simulink library "ee_lib_for_power_systems"  (R2025b)
settings: Solver=VariableStepAuto  StartTime=0.0  StopTime=10.0  RelTol=1e-3
undefined symbols (not defined in the file or in its folder): Alt_PolePair_Count, C3m, DiscIntNeverNeededParam, Enum1to12, L3m, Total_SM_Inertia, Ts
libraries: fl_lib x291, nesl_utility x219, ee_lib x139, custom_lib x12, $bdroot x10, ee_sl_lib x3, eeTransforms x2, eeGeneralControl x1, simulink x1
(part 10 of 11)

== system ee_lib_for_power_systems/Network Components/Bergeron Line (Pos-Zero)/tau ==
  u : Inport
  Delay : Delay  DelayLength=floor(tau(1)/Ts)  SampleTime=Ts
  Delay1 : Delay  DelayLength=floor(tau(2)/Ts)  SampleTime=Ts
  Delay2 : Delay  DelayLength=floor(tau(3)/Ts)  SampleTime=Ts
  Gain : Gain  Gain=[[tau/Ts - floor(tau/Ts)]' [tau/Ts - floor(tau/Ts)]']
  Matrix Concatenate : Concatenate  NumInputs=3  Mode=Multidimensional array  ConcatenateDimension=2
  Reshape : Reshape
  Selector : Selector  InputPortWidth=6  IndexOptions=Index vector (dialog)  Indices=[1 4]  OutputSizes=1
  Selector1 : Selector  InputPortWidth=6  IndexOptions=Index vector (dialog)  Indices=[2 5]  OutputSizes=1
  Selector2 : Selector  InputPortWidth=6  IndexOptions=Index vector (dialog)  Indices=[3 6]  OutputSizes=1
  Sum : Sum  Inputs=|-+
  Sum1 : Sum  Inputs=+-|
  Transpose : Math  Operator=transpose
  Unit Delay2 : UnitDelay  SampleTime=Ts  HasFrameUpgradeWarning=on
  y : Outport
  wires:
  Sum1/1 -> y/1
  Gain/1 -> Sum1/2
  Sum/1 -> Gain/1
  u/1 -> Reshape/1
  Unit Delay2/1 -> Sum/1
  Matrix Concatenate/1 -> Transpose/1
  Delay1/1 -> Matrix Concatenate/2
  Delay/1 -> Matrix Concatenate/1
  Delay2/1 -> Matrix Concatenate/3
  Selector/1 -> Delay/1
  Selector1/1 -> Delay1/1
  Reshape/1 -> Selector2/1, Selector/1, Selector1/1
  Selector2/1 -> Delay2/1
  Transpose/1 -> Sum/2, Unit Delay2/1, Sum1/1

== system ee_lib_for_power_systems/Network Components/Bergeron Line (Pos-Zero)/tau  ==
  u : Inport
  Delay : Delay  DelayLength=floor(tau(1)/Ts)  SampleTime=Ts
  Delay1 : Delay  DelayLength=floor(tau(2)/Ts)  SampleTime=Ts
  Delay2 : Delay  DelayLength=floor(tau(3)/Ts)  SampleTime=Ts
  Gain : Gain  Gain=[[tau/Ts - floor(tau/Ts)]' [tau/Ts - floor(tau/Ts)]']
  Matrix Concatenate : Concatenate  NumInputs=3  Mode=Multidimensional array  ConcatenateDimension=2
  Reshape : Reshape
  Selector : Selector  InputPortWidth=6  IndexOptions=Index vector (dialog)  Indices=[1 4]  OutputSizes=1
  Selector1 : Selector  InputPortWidth=6  IndexOptions=Index vector (dialog)  Indices=[2 5]  OutputSizes=1
  Selector2 : Selector  InputPortWidth=6  IndexOptions=Index vector (dialog)  Indices=[3 6]  OutputSizes=1
  Sum : Sum  Inputs=|-+
  Sum1 : Sum  Inputs=+-|
  Transpose : Math  Operator=transpose
  Unit Delay2 : UnitDelay  SampleTime=Ts  HasFrameUpgradeWarning=on
  y : Outport
  wires:
  Transpose/1 -> Sum1/1, Unit Delay2/1, Sum/2
  Selector2/1 -> Delay2/1
  Reshape/1 -> Selector1/1, Selector/1, Selector2/1
  Selector1/1 -> Delay1/1
  Selector/1 -> Delay/1
  Delay2/1 -> Matrix Concatenate/3
  Delay/1 -> Matrix Concatenate/1
  Delay1/1 -> Matrix Concatenate/2
  Matrix Concatenate/1 -> Transpose/1
  Unit Delay2/1 -> Sum/1
  u/1 -> Reshape/1
  Sum/1 -> Gain/1
  Gain/1 -> Sum1/2
  Sum1/1 -> y/1

== system ee_lib_for_power_systems/Network Components/Bergeron Line (single-phase)/tau ==
  u : Inport
  Delay : Delay  DelayLength=floor(tau/Ts)  SampleTime=Ts
  Gain : Gain  Gain=tau/Ts - floor(tau/Ts)
  Sum : Sum  Inputs=|-+
  Sum1 : Sum  Inputs=+-|
  Unit Delay2 : UnitDelay  SampleTime=Ts  HasFrameUpgradeWarning=on
  y : Outport
  wires:
  Delay/1 -> Sum1/1, Unit Delay2/1, Sum/2
  u/1 -> Delay/1
  Unit Delay2/1 -> Sum/1
  Sum/1 -> Gain/1
  Gain/1 -> Sum1/2
  Sum1/1 -> y/1

== system ee_lib_for_power_systems/Network Components/Bergeron Line (single-phase)/tau  ==
  u : Inport
  Delay : Delay  DelayLength=floor(tau/Ts)  SampleTime=Ts
  Gain : Gain  Gain=tau/Ts - floor(tau/Ts)
  Sum : Sum  Inputs=|-+
  Sum1 : Sum  Inputs=+-|
  Unit Delay2 : UnitDelay  SampleTime=Ts  HasFrameUpgradeWarning=on
  y : Outport
  wires:
  Sum1/1 -> y/1
  Gain/1 -> Sum1/2
  Sum/1 -> Gain/1
  Unit Delay2/1 -> Sum/1
  u/1 -> Delay/1
  Delay/1 -> Sum/2, Unit Delay2/1, Sum1/1

== system ee_lib_for_power_systems/Network Components/Connector Bergeron Line (Pos-Zero)/tau ==
  u : Inport
  Delay : Delay  DelayLength=floor(tau(1)/Ts)  SampleTime=Ts
  Delay1 : Delay  DelayLength=floor(tau(2)/Ts)  SampleTime=Ts
  Delay2 : Delay  DelayLength=floor(tau(3)/Ts)  SampleTime=Ts
  Gain : Gain  Gain=[tau/Ts - floor(tau/Ts)]'
  Matrix Concatenate : Concatenate  NumInputs=3  Mode=Multidimensional array  ConcatenateDimension=2
  Reshape : Reshape
  Selector : Selector  InputPortWidth=3  IndexOptions=Index vector (dialog)  Indices=1  OutputSizes=1
  Selector1 : Selector  InputPortWidth=3  IndexOptions=Index vector (dialog)  Indices=2  OutputSizes=1
  Selector2 : Selector  InputPortWidth=3  IndexOptions=Index vector (dialog)  Indices=3  OutputSizes=1
  Sum : Sum  Inputs=|-+
  Sum1 : Sum  Inputs=+-|
  Transpose : Math  Operator=transpose
  Unit Delay2 : UnitDelay  SampleTime=Ts  HasFrameUpgradeWarning=on
  y : Outport
  wires:
  Sum1/1 -> y/1
  Gain/1 -> Sum1/2
  Sum/1 -> Gain/1
  u/1 -> Reshape/1
  Unit Delay2/1 -> Sum/1
  Matrix Concatenate/1 -> Transpose/1
  Delay1/1 -> Matrix Concatenate/2
  Delay/1 -> Matrix Concatenate/1
  Delay2/1 -> Matrix Concatenate/3
  Selector/1 -> Delay/1
  Selector1/1 -> Delay1/1
  Reshape/1 -> Selector2/1, Selector1/1, Selector/1
  Selector2/1 -> Delay2/1
  Transpose/1 -> Sum/2, Unit Delay2/1, Sum1/1

== system ee_lib_for_power_systems/Network Components/Connector Bergeron Line (Pos-Zero)/tau  ==
  u : Inport
  Delay : Delay  DelayLength=floor(tau(1)/Ts)  SampleTime=Ts
  Delay1 : Delay  DelayLength=floor(tau(2)/Ts)  SampleTime=Ts
  Delay2 : Delay  DelayLength=floor(tau(3)/Ts)  SampleTime=Ts
  Gain : Gain  Gain=[tau/Ts - floor(tau/Ts)]'
  Matrix Concatenate : Concatenate  NumInputs=3  Mode=Multidimensional array  ConcatenateDimension=2
  Reshape : Reshape
  Selector : Selector  InputPortWidth=3  IndexOptions=Index vector (dialog)  Indices=1  OutputSizes=1
  Selector1 : Selector  InputPortWidth=3  IndexOptions=Index vector (dialog)  Indices=2  OutputSizes=1
  Selector2 : Selector  InputPortWidth=3  IndexOptions=Index vector (dialog)  Indices=3  OutputSizes=1
  Sum : Sum  Inputs=|-+
  Sum1 : Sum  Inputs=+-|
  Transpose : Math  Operator=transpose
  Unit Delay2 : UnitDelay  SampleTime=Ts  HasFrameUpgradeWarning=on
  y : Outport
  wires:
  Transpose/1 -> Sum1/1, Unit Delay2/1, Sum/2
  Selector2/1 -> Delay2/1
  Reshape/1 -> Selector/1, Selector1/1, Selector2/1
  Selector1/1 -> Delay1/1
  Selector/1 -> Delay/1
  Delay2/1 -> Matrix Concatenate/3
  Delay/1 -> Matrix Concatenate/1
  Delay1/1 -> Matrix Concatenate/2
  Matrix Concatenate/1 -> Transpose/1
  Unit Delay2/1 -> Sum/1
  u/1 -> Reshape/1
  Sum/1 -> Gain/1
  Gain/1 -> Sum1/2
  Sum1/1 -> y/1

== system ee_lib_for_power_systems/Network Components/Connector Bergeron Line (single-phase)/tau ==
  u : Inport
  Delay : Delay  DelayLength=floor(tau/Ts)  SampleTime=Ts
  Gain : Gain  Gain=tau/Ts - floor(tau/Ts)
  Sum : Sum  Inputs=|-+
  Sum1 : Sum  Inputs=+-|
  Unit Delay2 : UnitDelay  SampleTime=Ts  HasFrameUpgradeWarning=on
  y : Outport
  wires:
  Delay/1 -> Sum1/1, Unit Delay2/1, Sum/2
  u/1 -> Delay/1
  Unit Delay2/1 -> Sum/1
  Sum/1 -> Gain/1
  Gain/1 -> Sum1/2
  Sum1/1 -> y/1

== system ee_lib_for_power_systems/Network Components/Connector Bergeron Line (single-phase)/tau  ==
  u : Inport
  Delay : Delay  DelayLength=floor(tau/Ts)  SampleTime=Ts
  Gain : Gain  Gain=tau/Ts - floor(tau/Ts)
  Sum : Sum  Inputs=|-+
  Sum1 : Sum  Inputs=+-|
  Unit Delay2 : UnitDelay  SampleTime=Ts  HasFrameUpgradeWarning=on
  y : Outport
  wires:
  Sum1/1 -> y/1
  Gain/1 -> Sum1/2
  Sum/1 -> Gain/1
  Unit Delay2/1 -> Sum/1
  u/1 -> Delay/1
  Delay/1 -> Sum/2, Unit Delay2/1, Sum1/1

== system ee_lib_for_power_systems/Power Converters/Ideal Switch Basic Diode Two-Level/S1 ==
  note: orientation based on anti-parallel diode
  g : Inport
  Ideal Switch with Basic Diode : custom_lib/Ideal Switch with Basic Diode  Ron=Ron Ohm  Goff=Goff 1/Ohm  i_specify=off  i_priority=None  i=0 A  i_nominal_specify=off  i_nominal_value=1  i_nominal_unit=A  v_specify=off  v_priority=None  v=0 V  v_nominal_specify=off  v_nominal_value=1  v_nominal_unit=V
      settings: InternalSimscapePortConfiguration="{\"Bottom\":[],\"Left\":[{\"id\":\"p\",\"label\":\"+\",\"type\":\"foundation.electrical.electrical\"},{\"id\":\"vT\",\"label\":\"\",\"type\":\"input\"}],\"Right\":[{\"id\":\"n\",\"label\":\"-\",\"type\":\"foundation.electrical.electrical\"}],\"Top\":[]}"
  Simulink-PS Converter : nesl_utility/Simulink-PS Converter  PhysicalDomain=network_engine_domain  SubClassName=ps_input  LeftPortType=input  RightPortType=output  PseudoPeriodic=off  Frequencies=[]  NoiseDistribution=none  NoiseParameters=[]  Unit=1  AffineConversion=off  FilteringAndDerivatives=provide  SimscapeFilterOrder=1  InputFilterTimeConstant=0.001  UdotUserProvided=0
  + : PMIOPort  Side=Left
  - : PMIOPort  Port=2  Side=Right
  wires:
  g/1 -> Simulink-PS Converter/1
  connections:
  Ideal Switch with Basic Diode/LConn1 -- -/RConn1
  Ideal Switch with Basic Diode/RConn1 -- +/RConn1
  Ideal Switch with Basic Diode/LConn2 -- Simulink-PS Converter/RConn1

== system ee_lib_for_power_systems/Power Converters/Three-Level Average-Value/Subsystem ==
  open : Inport
  Current Sensor : fl_lib/Electrical/Electrical Sensors/Current Sensor
  Data Type Conversion : DataTypeConversion  SaturateOnIntegerOverflow=off  RndMeth=Floor
  Ideal Semiconductor Switch{,1..3} (4 alike) : ee_lib/Semiconductors & Converters/Ideal Semiconductor Switch  port_option=ee.enum.controlport.ps  Ron=1e-8 Ohm  Goff=1e-8 1/Ohm  Vth=0.5 V  diode_param=ee.enum.semiconductors.protectionDiode.external  diode_Vf=0.8 V  diode_Ron=0.001 Ohm  diode_Goff=1e-5 1/Ohm  diode_C=50e-9 F  diode_iRM=-235 A  diode_iF=300 A  diode_idot=-50 A/us  diode_trr_param=ee.enum.converters.diodetrr.trr_factor  diode_trr_factor=3  diode_trr=15 us  diode_Qrr=1500 s*uA
  NOT : Logic  Operator=NOT  IconShape=distinctive  AllPortsSameDT=off  OutDataTypeStr=boolean
  Simulink-PS Converter2 : nesl_utility/Simulink-PS Converter  PhysicalDomain=network_engine_domain  SubClassName=ps_input  LeftPortType=input  RightPortType=output  PseudoPeriodic=off  Frequencies=[]  NoiseDistribution=none  NoiseParameters=[]  Unit=1  AffineConversion=off  FilteringAndDerivatives=provide  SimscapeFilterOrder=1  InputFilterTimeConstant=0.001  UdotUserProvided=0
  Simulink-PS Converter3 : nesl_utility/Simulink-PS Converter  PhysicalDomain=network_engine_domain  SubClassName=ps_input  LeftPortType=input  RightPortType=output  PseudoPeriodic=off  Frequencies=[]  NoiseDistribution=none  NoiseParameters=[]  Unit=1  AffineConversion=off  FilteringAndDerivatives=provide  SimscapeFilterOrder=1  InputFilterTimeConstant=0.001  UdotUserProvided=0
  N : PMIOPort  Side=Left
  +1 : PMIOPort  Port=2  Side=Left
  +2 : PMIOPort  Port=3  Side=Left
  Iconn : PMIOPort  Port=4  Side=Right
  -1 : PMIOPort  Port=5  Side=Right
  -2 : PMIOPort  Port=6  Side=Right
  wires:
  open/1 -> Simulink-PS Converter2/1, NOT/1
  NOT/1 -> Data Type Conversion/1
  Data Type Conversion/1 -> Simulink-PS Converter3/1
  connections:
  Current Sensor/RConn2 -- Ideal Semiconductor Switch1/RConn1 -- Ideal Semiconductor Switch2/RConn2
  Iconn/RConn1 -- Current Sensor/RConn1
  Current Sensor/LConn1 -- N/RConn1
  Ideal Semiconductor Switch2/LConn1 -- Simulink-PS Converter3/RConn1 -- Ideal Semiconductor Switch1/LConn1
  +2/RConn1 -- Ideal Semiconductor Switch/RConn1 -- Ideal Semiconductor Switch1/RConn2
  Ideal Semiconductor Switch/LConn1 -- Simulink-PS Converter2/RConn1 -- Ideal Semiconductor Switch3/LConn1
  Ideal Semiconductor Switch/RConn2 -- +1/RConn1
  Ideal Semiconductor Switch3/RConn1 -- Ideal Semiconductor Switch2/RConn1 -- -2/RConn1
  -1/RConn1 -- Ideal Semiconductor Switch3/RConn2

== system ee_lib_for_power_systems/Power Converters/Three-Level Average-Value/Subsystem1 ==
  open : Inport
  g_h : Inport  Port=2
  Constant1 : Constant  Value=0  SampleTime=-1
  Switch : Switch  Criteria=u2 > Threshold  Threshold=0.5  SaturateOnIntegerOverflow=off  InputSameDT=off
  p : Outport
  wires:
  g_h/1 -> Switch/3
  open/1 -> Switch/2
  Constant1/1 -> Switch/1
  Switch/1 -> p/1

== system ee_lib_for_power_systems/Power Converters/Three-Level Average-Value/Subsystem2 ==
  p3 : Inport
  p2 : Inport  Port=2
  p1 : Inport  Port=3
  PS Add : fl_lib/Physical Signals/Functions/PS Add
  PS Add1 : fl_lib/Physical Signals/Functions/PS Add
  PS Gain : fl_lib/Physical Signals/Functions/PS Gain  gain=-1
  PS Product2 : fl_lib/Physical Signals/Functions/PS Product
  PS Product7 : fl_lib/Physical Signals/Functions/PS Product
  PS Product8 : fl_lib/Physical Signals/Functions/PS Product
  Simulink-PS Converter : nesl_utility/Simulink-PS Converter  PhysicalDomain=network_engine_domain  SubClassName=ps_input  LeftPortType=input  RightPortType=output  PseudoPeriodic=off  Frequencies=[]  NoiseDistribution=none  NoiseParameters=[]  Unit=1  AffineConversion=off  FilteringAndDerivatives=provide  SimscapeFilterOrder=1  InputFilterTimeConstant=0.001  UdotUserProvided=0
  Simulink-PS Converter1 : nesl_utility/Simulink-PS Converter  PhysicalDomain=network_engine_domain  SubClassName=ps_input  LeftPortType=input  RightPortType=output  PseudoPeriodic=off  Frequencies=[]  NoiseDistribution=none  NoiseParameters=[]  Unit=1  AffineConversion=off  FilteringAndDerivatives=provide  SimscapeFilterOrder=1  InputFilterTimeConstant=0.001  UdotUserProvided=0
  Simulink-PS Converter2 : nesl_utility/Simulink-PS Converter  PhysicalDomain=network_engine_domain  SubClassName=ps_input  LeftPortType=input  RightPortType=output  PseudoPeriodic=off  Frequencies=[]  NoiseDistribution=none  NoiseParameters=[]  Unit=1  AffineConversion=off  FilteringAndDerivatives=provide  SimscapeFilterOrder=1  InputFilterTimeConstant=0.001  UdotUserProvided=0
  IAP : PMIOPort  Side=Left
  IBP : PMIOPort  Port=2  Side=Left
  ICP : PMIOPort  Port=3  Side=Left
  IDCP : PMIOPort  Port=4  Side=Right
  wires:
  p3/1 -> Simulink-PS Converter2/1
  p2/1 -> Simulink-PS Converter1/1
  p1/1 -> Simulink-PS Converter/1
  connections:
  PS Gain/LConn1 -- PS Add1/RConn1
  PS Gain/RConn1 -- IDCP/RConn1
  PS Product2/LConn1 -- IAP/RConn1
  PS Add1/LConn1 -- PS Product8/RConn1
  PS Add1/LConn2 -- PS Add/RConn1
  Simulink-PS Converter/RConn1 -- PS Product2/LConn2
  PS Add/LConn2 -- PS Product2/RConn1
  PS Add/LConn1 -- PS Product7/RConn1
  PS Product8/LConn1 -- ICP/RConn1
  PS Product8/LConn2 -- Simulink-PS Converter2/RConn1
  PS Product7/LConn2 -- Simulink-PS Converter1/RConn1
  PS Product7/LConn1 -- IBP/RConn1

== system ee_lib_for_power_systems/Power Converters/Three-Level Average-Value/Subsystem4 ==
  p3 : Inport
  p2 : Inport  Port=2
  p1 : Inport  Port=3
  PS Add : fl_lib/Physical Signals/Functions/PS Add
  PS Add1 : fl_lib/Physical Signals/Functions/PS Add
  PS Gain : fl_lib/Physical Signals/Functions/PS Gain  gain=1
  PS Product2 : fl_lib/Physical Signals/Functions/PS Product
  PS Product7 : fl_lib/Physical Signals/Functions/PS Product
  PS Product8 : fl_lib/Physical Signals/Functions/PS Product
  Simulink-PS Converter : nesl_utility/Simulink-PS Converter  PhysicalDomain=network_engine_domain  SubClassName=ps_input  LeftPortType=input  RightPortType=output  PseudoPeriodic=off  Frequencies=[]  NoiseDistribution=none  NoiseParameters=[]  Unit=1  AffineConversion=off  FilteringAndDerivatives=provide  SimscapeFilterOrder=1  InputFilterTimeConstant=0.001  UdotUserProvided=0
  Simulink-PS Converter1 : nesl_utility/Simulink-PS Converter  PhysicalDomain=network_engine_domain  SubClassName=ps_input  LeftPortType=input  RightPortType=output  PseudoPeriodic=off  Frequencies=[]  NoiseDistribution=none  NoiseParameters=[]  Unit=1  AffineConversion=off  FilteringAndDerivatives=provide  SimscapeFilterOrder=1  InputFilterTimeConstant=0.001  UdotUserProvided=0
  Simulink-PS Converter2 : nesl_utility/Simulink-PS Converter  PhysicalDomain=network_engine_domain  SubClassName=ps_input  LeftPortType=input  RightPortType=output  PseudoPeriodic=off  Frequencies=[]  NoiseDistribution=none  NoiseParameters=[]  Unit=1  AffineConversion=off  FilteringAndDerivatives=provide  SimscapeFilterOrder=1  InputFilterTimeConstant=0.001  UdotUserProvided=0
  IAN : PMIOPort  Side=Left
  IBN : PMIOPort  Port=2  Side=Left
  ICN : PMIOPort  Port=3  Side=Left
  IDCN : PMIOPort  Port=4  Side=Right
  wires:
  p1/1 -> Simulink-PS Converter/1
  p2/1 -> Simulink-PS Converter1/1
  p3/1 -> Simulink-PS Converter2/1
  connections:
  PS Product7/LConn1 -- IBN/RConn1
  PS Product7/LConn2 -- Simulink-PS Converter1/RConn1
  PS Product8/LConn2 -- Simulink-PS Converter2/RConn1
  PS Product8/LConn1 -- ICN/RConn1
  PS Add/LConn1 -- PS Product7/RConn1
  PS Add/LConn2 -- PS Product2/RConn1
  Simulink-PS Converter/RConn1 -- PS Product2/LConn2
  PS Add1/LConn2 -- PS Add/RConn1
  PS Add1/LConn1 -- PS Product8/RConn1
  PS Product2/LConn1 -- IAN/RConn1
  PS Gain/RConn1 -- IDCN/RConn1
  PS Add1/RConn1 -- PS Gain/LConn1

== system ee_lib_for_power_systems/Power Converters/Three-Level NPC Average-Switch with Diodes/IDCN ==
  p3 : Inport
  p2 : Inport  Port=2
  p1 : Inport  Port=3
  PS Add : fl_lib/Physical Signals/Functions/PS Add
  PS Add1 : fl_lib/Physical Signals/Functions/PS Add
  PS Gain : fl_lib/Physical Signals/Functions/PS Gain  gain=1
  PS Product1 : fl_lib/Physical Signals/Functions/PS Product
  PS Product2 : fl_lib/Physical Signals/Functions/PS Product
  PS Product3 : fl_lib/Physical Signals/Functions/PS Product
  Simulink-PS Converter1 : nesl_utility/Simulink-PS Converter  PhysicalDomain=network_engine_domain  SubClassName=ps_input  LeftPortType=input  RightPortType=output  PseudoPeriodic=off  Frequencies=[]  NoiseDistribution=none  NoiseParameters=[]  Unit=1  AffineConversion=off  FilteringAndDerivatives=zero  SimscapeFilterOrder=1  InputFilterTimeConstant=0.001  UdotUserProvided=0
  Simulink-PS Converter2 : nesl_utility/Simulink-PS Converter  PhysicalDomain=network_engine_domain  SubClassName=ps_input  LeftPortType=input  RightPortType=output  PseudoPeriodic=off  Frequencies=[]  NoiseDistribution=none  NoiseParameters=[]  Unit=1  AffineConversion=off  FilteringAndDerivatives=zero  SimscapeFilterOrder=1  InputFilterTimeConstant=0.001  UdotUserProvided=0
  Simulink-PS Converter3 : nesl_utility/Simulink-PS Converter  PhysicalDomain=network_engine_domain  SubClassName=ps_input  LeftPortType=input  RightPortType=output  PseudoPeriodic=off  Frequencies=[]  NoiseDistribution=none  NoiseParameters=[]  Unit=1  AffineConversion=off  FilteringAndDerivatives=zero  SimscapeFilterOrder=1  InputFilterTimeConstant=0.001  UdotUserProvided=0
  IAN : PMIOPort  Side=Left
  IBN : PMIOPort  Port=2  Side=Left
  ICN : PMIOPort  Port=3  Side=Left
  IDCN : PMIOPort  Port=4  Side=Right
  wires:
  p2/1 -> Simulink-PS Converter1/1
  p3/1 -> Simulink-PS Converter3/1
  p1/1 -> Simulink-PS Converter2/1
  connections:
  PS Product1/RConn1 -- PS Add1/LConn1
  PS Add1/RConn1 -- PS Add/LConn2
  PS Product3/RConn1 -- PS Add/LConn1
  IDCN/RConn1 -- PS Gain/RConn1
  PS Gain/LConn1 -- PS Add/RConn1
  PS Product2/RConn1 -- PS Add1/LConn2
  IAN/RConn1 -- PS Product2/LConn1
  IBN/RConn1 -- PS Product1/LConn1
  ICN/RConn1 -- PS Product3/LConn1
  Simulink-PS Converter2/RConn1 -- PS Product2/LConn2
  Simulink-PS Converter1/RConn1 -- PS Product1/LConn2
  Simulink-PS Converter3/RConn1 -- PS Product3/LConn2

== system ee_lib_for_power_systems/Power Converters/Three-Level NPC Average-Switch with Diodes/IDCP ==
  p3 : Inport
  p2 : Inport  Port=2
  p1 : Inport  Port=3
  PS Add : fl_lib/Physical Signals/Functions/PS Add
  PS Add1 : fl_lib/Physical Signals/Functions/PS Add
  PS Gain : fl_lib/Physical Signals/Functions/PS Gain  gain=-1
  PS Product : fl_lib/Physical Signals/Functions/PS Product
  PS Product1 : fl_lib/Physical Signals/Functions/PS Product
  PS Product2 : fl_lib/Physical Signals/Functions/PS Product
  Simulink-PS Converter : nesl_utility/Simulink-PS Converter  PhysicalDomain=network_engine_domain  SubClassName=ps_input  LeftPortType=input  RightPortType=output  PseudoPeriodic=off  Frequencies=[]  NoiseDistribution=none  NoiseParameters=[]  Unit=1  AffineConversion=off  FilteringAndDerivatives=zero  SimscapeFilterOrder=1  InputFilterTimeConstant=0.001  UdotUserProvided=0
  Simulink-PS Converter1 : nesl_utility/Simulink-PS Converter  PhysicalDomain=network_engine_domain  SubClassName=ps_input  LeftPortType=input  RightPortType=output  PseudoPeriodic=off  Frequencies=[]  NoiseDistribution=none  NoiseParameters=[]  Unit=1  AffineConversion=off  FilteringAndDerivatives=zero  SimscapeFilterOrder=1  InputFilterTimeConstant=0.001  UdotUserProvided=0
  Simulink-PS Converter2 : nesl_utility/Simulink-PS Converter  PhysicalDomain=network_engine_domain  SubClassName=ps_input  LeftPortType=input  RightPortType=output  PseudoPeriodic=off  Frequencies=[]  NoiseDistribution=none  NoiseParameters=[]  Unit=1  AffineConversion=off  FilteringAndDerivatives=zero  SimscapeFilterOrder=1  InputFilterTimeConstant=0.001  UdotUserProvided=0
  IAP : PMIOPort  Side=Left
  IBP : PMIOPort  Port=2  Side=Left
  ICP : PMIOPort  Port=3  Side=Left
  IDCP : PMIOPort  Port=4  Side=Right
  wires:
  p1/1 -> Simulink-PS Converter/1
  p3/1 -> Simulink-PS Converter2/1
  p2/1 -> Simulink-PS Converter1/1
  connections:
  PS Gain/RConn1 -- IDCP/RConn1
  PS Add/RConn1 -- PS Gain/LConn1
  PS Product2/RConn1 -- PS Add/LConn1
  PS Add1/RConn1 -- PS Add/LConn2
  PS Product1/RConn1 -- PS Add1/LConn1
  PS Product/RConn1 -- PS Add1/LConn2
  IAP/RConn1 -- PS Product/LConn1
  IBP/RConn1 -- PS Product1/LConn1
  ICP/RConn1 -- PS Product2/LConn1
  Simulink-PS Converter/RConn1 -- PS Product/LConn2
  Simulink-PS Converter1/RConn1 -- PS Product1/LConn2
  Simulink-PS Converter2/RConn1 -- PS Product2/LConn2

== system ee_lib_for_power_systems/Power Converters/Three-Level NPC Average-Switch with Diodes/IN ==
  p3 : Inport
  p2 : Inport  Port=2
  p1 : Inport  Port=3
  PS Add : fl_lib/Physical Signals/Functions/PS Add
  PS Add1 : fl_lib/Physical Signals/Functions/PS Add
  PS Product : fl_lib/Physical Signals/Functions/PS Product
  PS Product1 : fl_lib/Physical Signals/Functions/PS Product
  PS Product2 : fl_lib/Physical Signals/Functions/PS Product
  Simulink-PS Converter : nesl_utility/Simulink-PS Converter  PhysicalDomain=network_engine_domain  SubClassName=ps_input  LeftPortType=input  RightPortType=output  PseudoPeriodic=off  Frequencies=[]  NoiseDistribution=none  NoiseParameters=[]  Unit=1  AffineConversion=off  FilteringAndDerivatives=zero  SimscapeFilterOrder=1  InputFilterTimeConstant=0.001  UdotUserProvided=0
  Simulink-PS Converter1 : nesl_utility/Simulink-PS Converter  PhysicalDomain=network_engine_domain  SubClassName=ps_input  LeftPortType=input  RightPortType=output  PseudoPeriodic=off  Frequencies=[]  NoiseDistribution=none  NoiseParameters=[]  Unit=1  AffineConversion=off  FilteringAndDerivatives=zero  SimscapeFilterOrder=1  InputFilterTimeConstant=0.001  UdotUserProvided=0
  Simulink-PS Converter2 : nesl_utility/Simulink-PS Converter  PhysicalDomain=network_engine_domain  SubClassName=ps_input  LeftPortType=input  RightPortType=output  PseudoPeriodic=off  Frequencies=[]  NoiseDistribution=none  NoiseParameters=[]  Unit=1  AffineConversion=off  FilteringAndDerivatives=zero  SimscapeFilterOrder=1  InputFilterTimeConstant=0.001  UdotUserProvided=0
  IA : PMIOPort  Side=Left
  IB : PMIOPort  Port=2  Side=Left
  IC : PMIOPort  Port=3  Side=Left
  IN : PMIOPort  Port=4  Side=Right
  wires:
  p1/1 -> Simulink-PS Converter/1
  p2/1 -> Simulink-PS Converter1/1
  p3/1 -> Simulink-PS Converter2/1
  connections:
  PS Product/RConn1 -- PS Add1/LConn2
  PS Product1/RConn1 -- PS Add1/LConn1
  PS Add1/RConn1 -- PS Add/LConn2
  PS Product2/RConn1 -- PS Add/LConn1
  PS Add/RConn1 -- IN/RConn1
  IA/RConn1 -- PS Product/LConn1
  IB/RConn1 -- PS Product1/LConn1
  IC/RConn1 -- PS Product2/LConn1
  Simulink-PS Converter/RConn1 -- PS Product/LConn2
  Simulink-PS Converter1/RConn1 -- PS Product1/LConn2
  Simulink-PS Converter2/RConn1 -- PS Product2/LConn2

== system ee_lib_for_power_systems/Power Converters/Three-Level NPC Average-Switch with Diodes/Subsystem ==
  open : Inport
  Data Type Conversion : DataTypeConversion  SaturateOnIntegerOverflow=off  RndMeth=Floor
  Ideal Semiconductor Switch{,1..3} (4 alike) : ee_lib/Semiconductors & Converters/Ideal Semiconductor Switch  port_option=ee.enum.controlport.ps  Ron=Ron Ohm  Goff=Goff 1/Ohm  Vth=0.5 V  diode_param=ee.enum.semiconductors.protectionDiode.external  diode_Vf=0.8 V  diode_Ron=0.001 Ohm  diode_Goff=1e-5 1/Ohm  diode_C=50e-9 F  diode_iRM=-235 A  diode_iF=300 A  diode_idot=-50 A/us  diode_trr_param=ee.enum.converters.diodetrr.trr_factor  diode_trr_factor=3  diode_trr=15 us  diode_Qrr=1500 s*uA
  NOT : Logic  Operator=NOT  IconShape=distinctive  AllPortsSameDT=off  OutDataTypeStr=boolean
  Simulink-PS Converter2 : nesl_utility/Simulink-PS Converter  PhysicalDomain=network_engine_domain  SubClassName=ps_input  LeftPortType=input  RightPortType=output  PseudoPeriodic=off  Frequencies=[]  NoiseDistribution=none  NoiseParameters=[]  Unit=1  AffineConversion=off  FilteringAndDerivatives=provide  SimscapeFilterOrder=1  InputFilterTimeConstant=0.001  UdotUserProvided=0
  Simulink-PS Converter3 : nesl_utility/Simulink-PS Converter  PhysicalDomain=network_engine_domain  SubClassName=ps_input  LeftPortType=input  RightPortType=output  PseudoPeriodic=off  Frequencies=[]  NoiseDistribution=none  NoiseParameters=[]  Unit=1  AffineConversion=off  FilteringAndDerivatives=provide  SimscapeFilterOrder=1  InputFilterTimeConstant=0.001  UdotUserProvided=0
  N : PMIOPort  Side=Left
  +1 : PMIOPort  Port=2  Side=Left
  +2 : PMIOPort  Port=3  Side=Left
  -1 : PMIOPort  Port=4  Side=Right
  -2 : PMIOPort  Port=5  Side=Right
  wires:
  open/1 -> Simulink-PS Converter2/1, NOT/1
  NOT/1 -> Data Type Conversion/1
  Data Type Conversion/1 -> Simulink-PS Converter3/1
  connections:
  Ideal Semiconductor Switch2/RConn2 -- Ideal Semiconductor Switch3/RConn1 -- N/RConn1
  Ideal Semiconductor Switch2/LConn1 -- Ideal Semiconductor Switch3/LConn1 -- Simulink-PS Converter3/RConn1
  +2/RConn1 -- Ideal Semiconductor Switch3/RConn2 -- Ideal Semiconductor Switch/RConn1
  Ideal Semiconductor Switch/LConn1 -- Ideal Semiconductor Switch1/LConn1 -- Simulink-PS Converter2/RConn1
  Ideal Semiconductor Switch/RConn2 -- +1/RConn1
  Ideal Semiconductor Switch1/RConn1 -- Ideal Semiconductor Switch2/RConn1 -- -2/RConn1
  Ideal Semiconductor Switch1/RConn2 -- -1/RConn1

== system ee_lib_for_power_systems/Power Converters/Three-Level NPC Average-Switch with Diodes/Subsystem1 ==
  Voltage Sensor : fl_lib/Electrical/Electrical Sensors/Voltage Sensor
  + : PMIOPort  Side=Left
  - : PMIOPort  Port=2  Side=Right
  vmeas : PMIOPort  Port=3  Side=Right
  connections:
  vmeas/RConn1 -- Voltage Sensor/RConn1
  Voltage Sensor/LConn1 -- +/RConn1
  Voltage Sensor/RConn2 -- -/RConn1

== system ee_lib_for_power_systems/Power Converters/Three-Level NPC Average-Switch with Diodes/Subsystem11 ==
  open : Inport
  g_h : Inport  Port=2
  Constant1 : Constant  Value=[0 0 0]  SampleTime=-1
  Switch : Switch  Criteria=u2 > Threshold  Threshold=0.5  SaturateOnIntegerOverflow=off  InputSameDT=off
  p : Outport
  wires:
  Switch/1 -> p/1
  Constant1/1 -> Switch/1
  open/1 -> Switch/2
  g_h/1 -> Switch/3

== system ee_lib_for_power_systems/Power Converters/Three-Level NPC Average-Switch with Diodes/Subsystem3 ==
  Voltage Sensor : fl_lib/Electrical/Electrical Sensors/Voltage Sensor
  + : PMIOPort  Side=Left
  vmeas : PMIOPort  Port=2  Side=Left
  - : PMIOPort  Port=3  Side=Right
  connections:
  Voltage Sensor/RConn2 -- -/RConn1
  Voltage Sensor/LConn1 -- +/RConn1
  vmeas/RConn1 -- Voltage Sensor/RConn1

== system ee_lib_for_power_systems/Power Converters/Three-Level NPC Average-Switch with Diodes/Subsystem4 ==
  Voltage Sensor : fl_lib/Electrical/Electrical Sensors/Voltage Sensor
  + : PMIOPort  Side=Left
  - : PMIOPort  Port=2  Side=Right
  vmeas : PMIOPort  Port=3  Side=Right
  connections:
  Voltage Sensor/RConn2 -- -/RConn1
  Voltage Sensor/LConn1 -- +/RConn1
  vmeas/RConn1 -- Voltage Sensor/RConn1

== system ee_lib_for_power_systems/Power Converters/Three-Level NPC Average-Switch with Diodes/VN ==
  p1n : Inport
  p2n : Inport  Port=2
  p3n : Inport  Port=3
  PS Product : fl_lib/Physical Signals/Functions/PS Product
  PS Product1 : fl_lib/Physical Signals/Functions/PS Product
  PS Product2 : fl_lib/Physical Signals/Functions/PS Product
  Simulink-PS Converter7 : nesl_utility/Simulink-PS Converter  PhysicalDomain=network_engine_domain  SubClassName=ps_input  LeftPortType=input  RightPortType=output  PseudoPeriodic=off  Frequencies=[]  NoiseDistribution=none  NoiseParameters=[]  Unit=1  AffineConversion=off  FilteringAndDerivatives=provide  SimscapeFilterOrder=1  InputFilterTimeConstant=0.001  UdotUserProvided=0
  Simulink-PS Converter8 : nesl_utility/Simulink-PS Converter  PhysicalDomain=network_engine_domain  SubClassName=ps_input  LeftPortType=input  RightPortType=output  PseudoPeriodic=off  Frequencies=[]  NoiseDistribution=none  NoiseParameters=[]  Unit=1  AffineConversion=off  FilteringAndDerivatives=provide  SimscapeFilterOrder=1  InputFilterTimeConstant=0.001  UdotUserProvided=0
  Simulink-PS Converter9 : nesl_utility/Simulink-PS Converter  PhysicalDomain=network_engine_domain  SubClassName=ps_input  LeftPortType=input  RightPortType=output  PseudoPeriodic=off  Frequencies=[]  NoiseDistribution=none  NoiseParameters=[]  Unit=1  AffineConversion=off  FilteringAndDerivatives=provide  SimscapeFilterOrder=1  InputFilterTimeConstant=0.001  UdotUserProvided=0
  vn : PMIOPort  Side=Left
  v1n : PMIOPort  Port=2  Side=Right
  v2n : PMIOPort  Port=3  Side=Right
  v3n : PMIOPort  Port=4  Side=Right
  wires:
  p3n/1 -> Simulink-PS Converter9/1
  p1n/1 -> Simulink-PS Converter7/1
  p2n/1 -> Simulink-PS Converter8/1
  connections:
  v2n/RConn1 -- PS Product1/RConn1
  v1n/RConn1 -- PS Product/RConn1
  PS Product/LConn1 -- PS Product2/LConn1 -- PS Product1/LConn1 -- vn/RConn1
  v3n/RConn1 -- PS Product2/RConn1
  PS Product/LConn2 -- Simulink-PS Converter7/RConn1
  PS Product1/LConn2 -- Simulink-PS Converter8/RConn1
  PS Product2/LConn2 -- Simulink-PS Converter9/RConn1

== system ee_lib_for_power_systems/Power Converters/Three-Level NPC Average-Switch with Diodes/vref ==
  p : Inport
  PS Add2 : fl_lib/Physical Signals/Functions/PS Add
  PS Product : fl_lib/Physical Signals/Functions/PS Product
  Simulink-PS Converter : nesl_utility/Simulink-PS Converter  PhysicalDomain=network_engine_domain  SubClassName=ps_input  LeftPortType=input  RightPortType=output  PseudoPeriodic=off  Frequencies=[]  NoiseDistribution=none  NoiseParameters=[]  Unit=1  AffineConversion=off  FilteringAndDerivatives=zero  SimscapeFilterOrder=1  InputFilterTimeConstant=0.001  UdotUserProvided=0
  Vn : PMIOPort  Side=Left
  Vdc : PMIOPort  Port=2  Side=Left
  V : PMIOPort  Port=3  Side=Right
  wires:
  p/1 -> Simulink-PS Converter/1
  connections:
  PS Add2/LConn2 -- Vn/RConn1
  PS Product/RConn1 -- PS Add2/LConn1
  PS Add2/RConn1 -- V/RConn1
  Vdc/RConn1 -- PS Product/LConn2
  Simulink-PS Converter/RConn1 -- PS Product/LConn1

== system ee_lib_for_power_systems/Power Converters/Three-Level NPC Average-Switch with Diodes/vref1 ==
  p : Inport
  PS Add2 : fl_lib/Physical Signals/Functions/PS Add
  PS Product : fl_lib/Physical Signals/Functions/PS Product
  Simulink-PS Converter : nesl_utility/Simulink-PS Converter  PhysicalDomain=network_engine_domain  SubClassName=ps_input  LeftPortType=input  RightPortType=output  PseudoPeriodic=off  Frequencies=[]  NoiseDistribution=none  NoiseParameters=[]  Unit=1  AffineConversion=off  FilteringAndDerivatives=zero  SimscapeFilterOrder=1  InputFilterTimeConstant=0.001  UdotUserProvided=0
  Vn : PMIOPort  Side=Left
  Vdc : PMIOPort  Port=2  Side=Left
  V : PMIOPort  Port=3  Side=Right
  wires:
  p/1 -> Simulink-PS Converter/1
  connections:
  Simulink-PS Converter/RConn1 -- PS Product/LConn1
  Vdc/RConn1 -- PS Product/LConn2
  PS Add2/RConn1 -- V/RConn1
  PS Product/RConn1 -- PS Add2/LConn1
  PS Add2/LConn2 -- Vn/RConn1

== system ee_lib_for_power_systems/Power Converters/Three-Level NPC Average-Switch with Diodes/vref2 ==
  p : Inport
  PS Add2 : fl_lib/Physical Signals/Functions/PS Add
  PS Product : fl_lib/Physical Signals/Functions/PS Product
  Simulink-PS Converter : nesl_utility/Simulink-PS Converter  PhysicalDomain=network_engine_domain  SubClassName=ps_input  LeftPortType=input  RightPortType=output  PseudoPeriodic=off  Frequencies=[]  NoiseDistribution=none  NoiseParameters=[]  Unit=1  AffineConversion=off  FilteringAndDerivatives=zero  SimscapeFilterOrder=1  InputFilterTimeConstant=0.001  UdotUserProvided=0
  Vn : PMIOPort  Side=Left
  Vdc : PMIOPort  Port=2  Side=Left
  V : PMIOPort  Port=3  Side=Right
  wires:
  p/1 -> Simulink-PS Converter/1
  connections:
  PS Add2/LConn2 -- Vn/RConn1
  PS Product/RConn1 -- PS Add2/LConn1
  PS Add2/RConn1 -- V/RConn1
  Vdc/RConn1 -- PS Product/LConn2
  Simulink-PS Converter/RConn1 -- PS Product/LConn1

== system ee_lib_for_power_systems/Power Converters/Three-Level NPC Average-Switch with Diodes/vref3 ==
  p : Inport
  PS Add2 : fl_lib/Physical Signals/Functions/PS Add
  PS Product : fl_lib/Physical Signals/Functions/PS Product
  Simulink-PS Converter : nesl_utility/Simulink-PS Converter  PhysicalDomain=network_engine_domain  SubClassName=ps_input  LeftPortType=input  RightPortType=output  PseudoPeriodic=off  Frequencies=[]  NoiseDistribution=none  NoiseParameters=[]  Unit=1  AffineConversion=off  FilteringAndDerivatives=zero  SimscapeFilterOrder=1  InputFilterTimeConstant=0.001  UdotUserProvided=0
  Vn : PMIOPort  Side=Left
  V : PMIOPort  Port=2  Side=Right
  Vdc : PMIOPort  Port=3  Side=Right
  wires:
  p/1 -> Simulink-PS Converter/1
  connections:
  PS Add2/RConn1 -- V/RConn1
  PS Product/RConn1 -- PS Add2/LConn1
  PS Add2/LConn2 -- Vn/RConn1
  Vdc/RConn1 -- PS Product/LConn2
  Simulink-PS Converter/RConn1 -- PS Product/LConn1

== system ee_lib_for_power_systems/Power Converters/Three-Level NPC Average-Switch with Diodes/vref4 ==
  p : Inport
  PS Add2 : fl_lib/Physical Signals/Functions/PS Add
  PS Product : fl_lib/Physical Signals/Functions/PS Product
  Simulink-PS Converter : nesl_utility/Simulink-PS Converter  PhysicalDomain=network_engine_domain  SubClassName=ps_input  LeftPortType=input  RightPortType=output  PseudoPeriodic=off  Frequencies=[]  NoiseDistribution=none  NoiseParameters=[]  Unit=1  AffineConversion=off  FilteringAndDerivatives=zero  SimscapeFilterOrder=1  InputFilterTimeConstant=0.001  UdotUserProvided=0
  Vn : PMIOPort  Side=Left
  V : PMIOPort  Port=2  Side=Right
  Vdc : PMIOPort  Port=3  Side=Right
  wires:
  p/1 -> Simulink-PS Converter/1
  connections:
  Simulink-PS Converter/RConn1 -- PS Product/LConn1
  Vdc/RConn1 -- PS Product/LConn2
  PS Add2/LConn2 -- Vn/RConn1
  PS Product/RConn1 -- PS Add2/LConn1
  PS Add2/RConn1 -- V/RConn1

== system ee_lib_for_power_systems/Power Converters/Three-Level NPC Average-Switch with Diodes/vref5 ==
  p : Inport
  PS Add2 : fl_lib/Physical Signals/Functions/PS Add
  PS Product : fl_lib/Physical Signals/Functions/PS Product
  Simulink-PS Converter : nesl_utility/Simulink-PS Converter  PhysicalDomain=network_engine_domain  SubClassName=ps_input  LeftPortType=input  RightPortType=output  PseudoPeriodic=off  Frequencies=[]  NoiseDistribution=none  NoiseParameters=[]  Unit=1  AffineConversion=off  FilteringAndDerivatives=zero  SimscapeFilterOrder=1  InputFilterTimeConstant=0.001  UdotUserProvided=0
  Vn : PMIOPort  Side=Left
  V : PMIOPort  Port=2  Side=Right
  Vdc : PMIOPort  Port=3  Side=Right
  wires:
  p/1 -> Simulink-PS Converter/1
  connections:
  PS Add2/RConn1 -- V/RConn1
  PS Product/RConn1 -- PS Add2/LConn1
  PS Add2/LConn2 -- Vn/RConn1
  Vdc/RConn1 -- PS Product/LConn2
  Simulink-PS Converter/RConn1 -- PS Product/LConn1

== system ee_lib_for_power_systems/Power Converters/Thyristor Bridge (Ideal)/S1 ==
  G : Inport
  Ideal Thyristor : custom_lib/Ideal Thyristor  Vf=Vf V  Ron=Ron Ohm  Goff=Goff 1/Ohm  i_specify=off  i_priority=None  i=0 A  i_nominal_specify=off  i_nominal_value=1  i_nominal_unit=A  v_specify=off  v_priority=None  v=0 V  v_nominal_specify=off  v_nominal_value=1  v_nominal_unit=V  x_specify=off  x_priority=None  x=0.0  x_nominal_specify=off  x_nominal_value=1  x_nominal_unit=1  y_specify=off  y_priority=None  y=0.0  y_nominal_specify=off  y_nominal_value=1  y_nominal_unit=1
      settings: InternalSimscapePortConfiguration="{\"Bottom\":[],\"Left\":[{\"id\":\"p\",\"label\":\"+\",\"type\":\"foundation.electrical.electrical\"},{\"id\":\"g\",\"label\":\"g\",\"type\":\"input\"}],\"Right\":[{\"id\":\"n\",\"label\":\"-\",\"type\":\"foundation.electrical.electrical\"}],\"Top\":[]}"
  Simulink-PS Converter : nesl_utility/Simulink-PS Converter  PhysicalDomain=network_engine_domain  SubClassName=ps_input  LeftPortType=input  RightPortType=output  PseudoPeriodic=off  Frequencies=[]  NoiseDistribution=none  NoiseParameters=[]  Unit=1  AffineConversion=off  FilteringAndDerivatives=provide  SimscapeFilterOrder=1  InputFilterTimeConstant=0.001  UdotUserProvided=0
  + : PMIOPort  Side=Left
  - : PMIOPort  Port=2  Side=Right
  wires:
  G/1 -> Simulink-PS Converter/1
  connections:
  Ideal Thyristor/LConn1 -- +/RConn1
  Ideal Thyristor/RConn1 -- -/RConn1
  Ideal Thyristor/LConn2 -- Simulink-PS Converter/RConn1

== system ee_lib_for_power_systems/Power Converters/Two-Level Average-Switch with Diodes/Subsystem ==
  open : Inport
  Data Type Conversion : DataTypeConversion  SaturateOnIntegerOverflow=off  RndMeth=Floor
  Ideal Semiconductor Switch : ee_lib/Semiconductors & Converters/Ideal Semiconductor Switch  port_option=ee.enum.controlport.ps  Ron=1e-5 Ohm  Goff=1e-5 1/Ohm  Vth=0.5 V  diode_param=ee.enum.semiconductors.protectionDiode.external  diode_Vf=0.8 V  diode_Ron=0.001 Ohm  diode_Goff=1e-5 1/Ohm  diode_C=50e-9 F  diode_iRM=-235 A  diode_iF=300 A  diode_idot=-50 A/us  diode_trr_param=ee.enum.converters.diodetrr.trr_factor  diode_trr_factor=3  diode_trr=15 us  diode_Qrr=1500 s*uA
  Ideal Semiconductor Switch1 : ee_lib/Semiconductors & Converters/Ideal Semiconductor Switch  port_option=ee.enum.controlport.ps  Ron=1e-5 Ohm  Goff=1e-5 1/Ohm  Vth=0.5 V  diode_param=ee.enum.semiconductors.protectionDiode.external  diode_Vf=0.8 V  diode_Ron=0.001 Ohm  diode_Goff=1e-5 1/Ohm  diode_C=50e-9 F  diode_iRM=-235 A  diode_iF=300 A  diode_idot=-50 A/us  diode_trr_param=ee.enum.converters.diodetrr.trr_factor  diode_trr_factor=3  diode_trr=15 us  diode_Qrr=1500 s*uA
  NOT : Logic  Operator=NOT  IconShape=distinctive  AllPortsSameDT=off  OutDataTypeStr=boolean
  Simulink-PS Converter2 : nesl_utility/Simulink-PS Converter  PhysicalDomain=network_engine_domain  SubClassName=ps_input  LeftPortType=input  RightPortType=output  PseudoPeriodic=off  Frequencies=[]  NoiseDistribution=none  NoiseParameters=[]  Unit=1  AffineConversion=off  FilteringAndDerivatives=provide  SimscapeFilterOrder=1  InputFilterTimeConstant=0.001  UdotUserProvided=0
  Simulink-PS Converter3 : nesl_utility/Simulink-PS Converter  PhysicalDomain=network_engine_domain  SubClassName=ps_input  LeftPortType=input  RightPortType=output  PseudoPeriodic=off  Frequencies=[]  NoiseDistribution=none  NoiseParameters=[]  Unit=1  AffineConversion=off  FilteringAndDerivatives=provide  SimscapeFilterOrder=1  InputFilterTimeConstant=0.001  UdotUserProvided=0
  +1 : PMIOPort  Side=Left
  +2 : PMIOPort  Port=2  Side=Left
  - : PMIOPort  Port=3  Side=Right
  wires:
  open/1 -> Simulink-PS Converter2/1, NOT/1
  NOT/1 -> Data Type Conversion/1
  Data Type Conversion/1 -> Simulink-PS Converter3/1
  connections:
  -/RConn1 -- Ideal Semiconductor Switch1/RConn1
  Ideal Semiconductor Switch1/LConn1 -- Simulink-PS Converter3/RConn1
  +2/RConn1 -- Ideal Semiconductor Switch/RConn1 -- Ideal Semiconductor Switch1/RConn2
  Ideal Semiconductor Switch/LConn1 -- Simulink-PS Converter2/RConn1
  Ideal Semiconductor Switch/RConn2 -- +1/RConn1

== system ee_lib_for_power_systems/Power Converters/Two-Level Average-Switch with Diodes/Subsystem1 ==
  open : Inport
  g_h : Inport  Port=2
  Constant1 : Constant  Value=0  SampleTime=-1
  Switch : Switch  Criteria=u2 > Threshold  Threshold=0.5  SaturateOnIntegerOverflow=off  InputSameDT=off
  p : Outport
  wires:
  g_h/1 -> Switch/3
  open/1 -> Switch/2
  Constant1/1 -> Switch/1
  Switch/1 -> p/1

== system ee_lib_for_power_systems/Power Converters/Two-Level Average-Switch with Diodes/Subsystem4 ==
  p1 : Inport
  p2 : Inport  Port=2
  p3 : Inport  Port=3
  PS Add{,1..4} (5 alike) : fl_lib/Physical Signals/Functions/PS Add
  PS Gain : fl_lib/Physical Signals/Functions/PS Gain  gain=-1
  PS Product{2,7..11} (6 alike) : fl_lib/Physical Signals/Functions/PS Product
  Simulink-PS Converter : nesl_utility/Simulink-PS Converter  PhysicalDomain=network_engine_domain  SubClassName=ps_input  LeftPortType=input  RightPortType=output  PseudoPeriodic=off  Frequencies=[]  NoiseDistribution=none  NoiseParameters=[]  Unit=1  AffineConversion=off  FilteringAndDerivatives=provide  SimscapeFilterOrder=1  InputFilterTimeConstant=0.001  UdotUserProvided=0
  Simulink-PS Converter1 : nesl_utility/Simulink-PS Converter  PhysicalDomain=network_engine_domain  SubClassName=ps_input  LeftPortType=input  RightPortType=output  PseudoPeriodic=off  Frequencies=[]  NoiseDistribution=none  NoiseParameters=[]  Unit=1  AffineConversion=off  FilteringAndDerivatives=provide  SimscapeFilterOrder=1  InputFilterTimeConstant=0.001  UdotUserProvided=0
  Simulink-PS Converter2 : nesl_utility/Simulink-PS Converter  PhysicalDomain=network_engine_domain  SubClassName=ps_input  LeftPortType=input  RightPortType=output  PseudoPeriodic=off  Frequencies=[]  NoiseDistribution=none  NoiseParameters=[]  Unit=1  AffineConversion=off  FilteringAndDerivatives=provide  SimscapeFilterOrder=1  InputFilterTimeConstant=0.001  UdotUserProvided=0
  IAN : PMIOPort  Side=Left
  IAP : PMIOPort  Port=2  Side=Left
  IBN : PMIOPort  Port=3  Side=Left
  IBP : PMIOPort  Port=4  Side=Left
  ICN : PMIOPort  Port=5  Side=Left
  ICP : PMIOPort  Port=6  Side=Left
  IDC : PMIOPort  Port=7  Side=Right
  wires:
  p1/1 -> Simulink-PS Converter/1
  p2/1 -> Simulink-PS Converter1/1
  p3/1 -> Simulink-PS Converter2/1
  connections:
  PS Product7/LConn1 -- IBN/RConn1
  PS Add2/LConn1 -- PS Product10/RConn1
  PS Add3/LConn1 -- PS Product11/RConn1
  PS Add3/LConn2 -- PS Add2/RConn1
  PS Add4/LConn2 -- PS Add1/RConn1
  PS Product7/LConn2 -- Simulink-PS Converter1/RConn1 -- PS Product10/LConn2
  PS Product8/LConn2 -- Simulink-PS Converter2/RConn1 -- PS Product11/LConn2
  PS Add2/LConn2 -- PS Product9/RConn1
  PS Product8/LConn1 -- ICN/RConn1
  PS Add/LConn1 -- PS Product7/RConn1
  PS Add/LConn2 -- PS Product2/RConn1
  PS Product9/LConn2 -- PS Product2/LConn2 -- Simulink-PS Converter/RConn1
  PS Add1/LConn2 -- PS Add/RConn1
  PS Product9/LConn1 -- IAP/RConn1
  PS Add1/LConn1 -- PS Product8/RConn1
  PS Gain/LConn1 -- PS Add3/RConn1
  PS Add4/LConn1 -- PS Gain/RConn1
  PS Add4/RConn1 -- IDC/RConn1
  PS Product2/LConn1 -- IAN/RConn1
  PS Product11/LConn1 -- ICP/RConn1
  PS Product10/LConn1 -- IBP/RConn1

== system ee_lib_for_power_systems/Power Converters/Two-Level Average-Value/Subsystem1 ==
  open : Inport
  g_h : Inport  Port=2
  Constant1 : Constant  Value=0  SampleTime=-1
  Switch : Switch  Criteria=u2 > Threshold  Threshold=0.5  SaturateOnIntegerOverflow=off  InputSameDT=off
  p : Outport
  wires:
  Switch/1 -> p/1
  Constant1/1 -> Switch/1
  open/1 -> Switch/2
  g_h/1 -> Switch/3

== system ee_lib_for_power_systems/Power Converters/Two-Level Average-Value/Subsystem2 ==
  p3 : Inport
  p2 : Inport  Port=2
  p1 : Inport  Port=3
  PS Add : fl_lib/Physical Signals/Functions/PS Add
  PS Add1 : fl_lib/Physical Signals/Functions/PS Add
  PS Gain : fl_lib/Physical Signals/Functions/PS Gain  gain=-1
  PS Product2 : fl_lib/Physical Signals/Functions/PS Product
  PS Product7 : fl_lib/Physical Signals/Functions/PS Product
  PS Product8 : fl_lib/Physical Signals/Functions/PS Product
  Simulink-PS Converter : nesl_utility/Simulink-PS Converter  PhysicalDomain=network_engine_domain  SubClassName=ps_input  LeftPortType=input  RightPortType=output  PseudoPeriodic=off  Frequencies=[]  NoiseDistribution=none  NoiseParameters=[]  Unit=1  AffineConversion=off  FilteringAndDerivatives=provide  SimscapeFilterOrder=1  InputFilterTimeConstant=0.001  UdotUserProvided=0
  Simulink-PS Converter1 : nesl_utility/Simulink-PS Converter  PhysicalDomain=network_engine_domain  SubClassName=ps_input  LeftPortType=input  RightPortType=output  PseudoPeriodic=off  Frequencies=[]  NoiseDistribution=none  NoiseParameters=[]  Unit=1  AffineConversion=off  FilteringAndDerivatives=provide  SimscapeFilterOrder=1  InputFilterTimeConstant=0.001  UdotUserProvided=0
  Simulink-PS Converter2 : nesl_utility/Simulink-PS Converter  PhysicalDomain=network_engine_domain  SubClassName=ps_input  LeftPortType=input  RightPortType=output  PseudoPeriodic=off  Frequencies=[]  NoiseDistribution=none  NoiseParameters=[]  Unit=1  AffineConversion=off  FilteringAndDerivatives=provide  SimscapeFilterOrder=1  InputFilterTimeConstant=0.001  UdotUserProvided=0
  IAP : PMIOPort  Side=Left
  IBP : PMIOPort  Port=2  Side=Left
  ICP : PMIOPort  Port=3  Side=Left
  IDCP : PMIOPort  Port=4  Side=Right
  wires:
  p1/1 -> Simulink-PS Converter/1
  p2/1 -> Simulink-PS Converter1/1
  p3/1 -> Simulink-PS Converter2/1
  connections:
  PS Product7/LConn1 -- IBP/RConn1
  PS Product7/LConn2 -- Simulink-PS Converter1/RConn1
  PS Product8/LConn2 -- Simulink-PS Converter2/RConn1
  PS Product8/LConn1 -- ICP/RConn1
  PS Add/LConn1 -- PS Product7/RConn1
  PS Add/LConn2 -- PS Product2/RConn1
  Simulink-PS Converter/RConn1 -- PS Product2/LConn2
  PS Add1/LConn2 -- PS Add/RConn1
  PS Add1/LConn1 -- PS Product8/RConn1
  PS Product2/LConn1 -- IAP/RConn1
  PS Gain/RConn1 -- IDCP/RConn1
  PS Gain/LConn1 -- PS Add1/RConn1

== system ee_lib_for_power_systems/Power Converters/Two-Level Average-Value/Subsystem3 ==
  open : Inport
  Data Type Conversion : DataTypeConversion  SaturateOnIntegerOverflow=off  RndMeth=Floor
  Ideal Semiconductor Switch : ee_lib/Semiconductors & Converters/Ideal Semiconductor Switch  port_option=ee.enum.controlport.ps  Ron=1e-5 Ohm  Goff=1e-5 1/Ohm  Vth=0.5 V  diode_param=ee.enum.semiconductors.protectionDiode.external  diode_Vf=0.8 V  diode_Ron=0.001 Ohm  diode_Goff=1e-5 1/Ohm  diode_C=50e-9 F  diode_iRM=-235 A  diode_iF=300 A  diode_idot=-50 A/us  diode_trr_param=ee.enum.converters.diodetrr.trr_factor  diode_trr_factor=3  diode_trr=15 us  diode_Qrr=1500 s*uA
  Ideal Semiconductor Switch1 : ee_lib/Semiconductors & Converters/Ideal Semiconductor Switch  port_option=ee.enum.controlport.ps  Ron=1e-5 Ohm  Goff=1e-5 1/Ohm  Vth=0.5 V  diode_param=ee.enum.semiconductors.protectionDiode.external  diode_Vf=0.8 V  diode_Ron=0.001 Ohm  diode_Goff=1e-5 1/Ohm  diode_C=50e-9 F  diode_iRM=-235 A  diode_iF=300 A  diode_idot=-50 A/us  diode_trr_param=ee.enum.converters.diodetrr.trr_factor  diode_trr_factor=3  diode_trr=15 us  diode_Qrr=1500 s*uA
  NOT : Logic  Operator=NOT  IconShape=distinctive  AllPortsSameDT=off  OutDataTypeStr=boolean
  Simulink-PS Converter2 : nesl_utility/Simulink-PS Converter  PhysicalDomain=network_engine_domain  SubClassName=ps_input  LeftPortType=input  RightPortType=output  PseudoPeriodic=off  Frequencies=[]  NoiseDistribution=none  NoiseParameters=[]  Unit=1  AffineConversion=off  FilteringAndDerivatives=provide  SimscapeFilterOrder=1  InputFilterTimeConstant=0.001  UdotUserProvided=0
  Simulink-PS Converter3 : nesl_utility/Simulink-PS Converter  PhysicalDomain=network_engine_domain  SubClassName=ps_input  LeftPortType=input  RightPortType=output  PseudoPeriodic=off  Frequencies=[]  NoiseDistribution=none  NoiseParameters=[]  Unit=1  AffineConversion=off  FilteringAndDerivatives=provide  SimscapeFilterOrder=1  InputFilterTimeConstant=0.001  UdotUserProvided=0
  +1 : PMIOPort  Side=Left
  +2 : PMIOPort  Port=2  Side=Left
  - : PMIOPort  Port=3  Side=Right
  wires:
  open/1 -> Simulink-PS Converter2/1, NOT/1
  NOT/1 -> Data Type Conversion/1
  Data Type Conversion/1 -> Simulink-PS Converter3/1
  connections:
  -/RConn1 -- Ideal Semiconductor Switch1/RConn1
  Ideal Semiconductor Switch1/LConn1 -- Simulink-PS Converter3/RConn1
  +2/RConn1 -- Ideal Semiconductor Switch/RConn1 -- Ideal Semiconductor Switch1/RConn2
  Ideal Semiconductor Switch/LConn1 -- Simulink-PS Converter2/RConn1
  Ideal Semiconductor Switch/RConn2 -- +1/RConn1
